simulink model "TosAeb"  (R2021a)
settings: Solver=FixedStepDiscrete  StartTime=0.0  StopTime=inf  FixedStep=0.01  RelTol=1e-3
undefined symbols (not defined in the file or in its folder): ACCModSts, AccSts1, AEB_ObjectMovingOrientation, AEB_ObjectMovingState, CN_BTCActive, CN_CurrentGearR, CN_CurrentGearR_DHT, CN_PTCActive, CN_VDCActive, CN_VSC_EspOff, CN_VSC_FCW_AEB_Error, CN_VSC_FCW_AEB_NoDispay, CN_VSC_FCW_AEB_PerfmDgdtn, CN_VSC_FCW_AEB_TempUnAvlbl, CN_VSC_VehStandstill, CNaccelAvoidMax, CNaccelAvoidMin, CNbrakePedalPress, CNcomfortStrLeftAccelMax, CNcomfortStrLeftAccelMin, CNcomfortStrRightAccelMax, CNcomfortStrRightAccelMin, CNDrvDoorOpen, CNDrvModeEco, CNDrvModeNorm, CNDrvModeOther, CNDrvModeSport, CNDrvSBRreminder, CNNull, CNobjInvalidID, CNPassDoorOpen, CNPassSBRreminder, CNPEBSAccelNegInf, CNPEBSAccelNull, CNPEBSTimeInf, CNPEBSTimeNull, CNSTOPMAX, CNSTOPNULL, CNsubjWidth, CNSwtOFF, CNSwtON, CNTTCMAX, CNTTCNULL, CNVehConfigAEBCycNone, CNVehConfigAEBNone, CNVehConfigAEBPedNone, CNVehConfigFCWNone, CNVehConfigJANone, CollisionSeverity, FALSE, FCWSnvty, JACycObjCalcInfoDefaultParams, JAPedObjCalcInfoDefaultParams, JAVehObjCalcInfoDefaultParams, latCycObjCalcInfoDefaultParams, latPedObjCalcInfoDefaultParams, longObjCalcInfoDefaultParams, ModSts, movingStatus, ObjectType ... (+1112)
libraries: simulink x3031, AEBSFunctionLib x50, Ta_Library x33, AEBSStateMachineLib x23, Mylibrary x12, simulink_extras x7, ObjSelect_lib x3, dspstat3 x2
(part 35 of 102)

== system TosAeb/FcwAebJa_Step_sys/ALG_AEBSystem/ALG_AEBController/AEB_VehicleStateCalcApp/VehSuppAborCondtionCalc/LatentWarningSuppAborConditonCalc/VehStateCalcLatentWarningSuppCalc ==
  AEB_VehResp : Inport
  FCW_AEB_VehFctSts : Inport  Port=2
  vehUnstableStateFlg : Inport  Port=3
  vehAssociatedSysStateSuppCond : Inport  Port=4
  "vehConfigFCW " : Inport  Port=5
  blockTimeWarning : Inport  Port=6
  Compare To Constant : simulink/Logic and Bit Operations/Compare To Constant  relop===  const=CNSwtOFF  OutDataTypeStr=boolean
  Compare To Constant1 : simulink/Logic and Bit Operations/Compare To Constant  relop===  const=CN_VSC_FCW_AEB_Error  OutDataTypeStr=boolean
  Compare To Constant2 : simulink/Logic and Bit Operations/Compare To Constant  relop===  const=CN_VSC_FCW_AEB_PerfmDgdtn  OutDataTypeStr=boolean
  Compare To Constant3 : simulink/Logic and Bit Operations/Compare To Constant  relop===  const=CNVehConfigFCWNone  OutDataTypeStr=boolean
  Compare To Constant4 : simulink/Logic and Bit Operations/Compare To Constant  relop=>  const=CNNull  OutDataTypeStr=boolean
  Compare To Constant5 : simulink/Logic and Bit Operations/Compare To Constant  relop===  const=TRUE  OutDataTypeStr=boolean
  Compare To Constant6 : simulink/Logic and Bit Operations/Compare To Constant  relop===  const=TRUE  OutDataTypeStr=boolean
  Compare To Constant7 : simulink/Logic and Bit Operations/Compare To Constant  relop===  const=CNSwtOFF  OutDataTypeStr=boolean
  Compare To Constant8 : simulink/Logic and Bit Operations/Compare To Constant  relop===  const=CN_VSC_FCW_AEB_TempUnAvlbl  OutDataTypeStr=boolean
  Constant : Constant  Value=P_AEB_InterSwt4LatentWarning
  Logical Operator : Logic  Operator=OR  Inputs=3  AllPortsSameDT=off  OutDataTypeStr=boolean
  Logical Operator1 : Logic  Operator=OR  Inputs=6  AllPortsSameDT=off  OutDataTypeStr=boolean
  Terminator : Terminator
  VSCvehLatentWarningSuppCond : Outport
  wires:
  Compare To Constant8/1 -> Logical Operator/3
  Compare To Constant1/1 -> Logical Operator/1
  Compare To Constant2/1 -> Logical Operator/2
  Compare To Constant7/1 -> Logical Operator1/6
  Constant/1 -> Compare To Constant7/1
  Compare To Constant6/1 -> Logical Operator1/3
  Compare To Constant5/1 -> Logical Operator1/4
  AEB_VehResp/1 -> Compare To Constant/1
  Compare To Constant/1 -> Logical Operator1/1
  Compare To Constant3/1 -> Logical Operator1/5
  vehAssociatedSysStateSuppCond/1 -> Compare To Constant5/1
  FCW_AEB_VehFctSts/1 -> Compare To Constant1/1, Compare To Constant8/1, Compare To Constant2/1
  blockTimeWarning/1 -> Compare To Constant4/1
  Logical Operator/1 -> Logical Operator1/2
  vehUnstableStateFlg/1 -> Compare To Constant6/1
  Logical Operator1/1 -> VSCvehLatentWarningSuppCond/1
  "vehConfigFCW "/1 -> Compare To Constant3/1
  Compare To Constant4/1 -> Terminator/1

== system TosAeb/FcwAebJa_Step_sys/ALG_AEBSystem/ALG_AEBController/AEB_VehicleStateCalcApp/VehSuppAborCondtionCalc/PreWarningSuppAborConditonCalc/VehStateCalcPreWarningAborCalc ==
  AEB_VehResp : Inport
  FCW_AEB_VehFctSts : Inport  Port=2
  vehAssociatedSysStateSuppCond : Inport  Port=3
  Compare To Constant : simulink/Logic and Bit Operations/Compare To Constant  relop===  const=CNSwtOFF  OutDataTypeStr=boolean
  Compare To Constant1 : simulink/Logic and Bit Operations/Compare To Constant  relop===  const=CN_VSC_FCW_AEB_Error  OutDataTypeStr=boolean
  Compare To Constant2 : simulink/Logic and Bit Operations/Compare To Constant  relop===  const=CN_VSC_FCW_AEB_PerfmDgdtn  OutDataTypeStr=boolean
  Compare To Constant3 : simulink/Logic and Bit Operations/Compare To Constant  relop===  const=TRUE  OutDataTypeStr=boolean
  Compare To Constant8 : simulink/Logic and Bit Operations/Compare To Constant  relop===  const=CN_VSC_FCW_AEB_TempUnAvlbl  OutDataTypeStr=boolean
  Logical Operator : Logic  Operator=OR  Inputs=3  AllPortsSameDT=off  OutDataTypeStr=boolean
  Logical Operator1 : Logic  Operator=OR  Inputs=3  AllPortsSameDT=off  OutDataTypeStr=boolean
  VSCvehPreWarningAborCond : Outport
  wires:
  Compare To Constant8/1 -> Logical Operator/3
  Compare To Constant1/1 -> Logical Operator/1
  Compare To Constant2/1 -> Logical Operator/2
  Compare To Constant3/1 -> Logical Operator1/3
  Logical Operator1/1 -> VSCvehPreWarningAborCond/1
  Logical Operator/1 -> Logical Operator1/2
  FCW_AEB_VehFctSts/1 -> Compare To Constant1/1, Compare To Constant2/1, Compare To Constant8/1
  vehAssociatedSysStateSuppCond/1 -> Compare To Constant3/1
  Compare To Constant/1 -> Logical Operator1/1
  AEB_VehResp/1 -> Compare To Constant/1

== system TosAeb/FcwAebJa_Step_sys/ALG_AEBSystem/ALG_AEBController/AEB_VehicleStateCalcApp/VehSuppAborCondtionCalc/PreWarningSuppAborConditonCalc/VehStateCalcPreWarningSuppCalc ==
  AEB_VehResp : Inport
  FCW_AEB_VehFctSts : Inport  Port=2
  vehUnstableStateFlg : Inport  Port=3
  vehAssociatedSysStateSuppCond : Inport  Port=4
  "vehConfigFCW " : Inport  Port=5
  blockTimeWarning : Inport  Port=6
  Compare To Constant : simulink/Logic and Bit Operations/Compare To Constant  relop===  const=CNSwtOFF  OutDataTypeStr=boolean
  Compare To Constant1 : simulink/Logic and Bit Operations/Compare To Constant  relop===  const=CN_VSC_FCW_AEB_Error  OutDataTypeStr=boolean
  Compare To Constant2 : simulink/Logic and Bit Operations/Compare To Constant  relop===  const=CN_VSC_FCW_AEB_PerfmDgdtn  OutDataTypeStr=boolean
  Compare To Constant3 : simulink/Logic and Bit Operations/Compare To Constant  relop===  const=CNVehConfigFCWNone  OutDataTypeStr=boolean
  Compare To Constant4 : simulink/Logic and Bit Operations/Compare To Constant  relop=>  const=CNNull  OutDataTypeStr=boolean
  Compare To Constant5 : simulink/Logic and Bit Operations/Compare To Constant  relop===  const=TRUE  OutDataTypeStr=boolean
  Compare To Constant6 : simulink/Logic and Bit Operations/Compare To Constant  relop===  const=TRUE  OutDataTypeStr=boolean
  Compare To Constant7 : simulink/Logic and Bit Operations/Compare To Constant  relop===  const=CNSwtOFF  OutDataTypeStr=boolean
  Compare To Constant8 : simulink/Logic and Bit Operations/Compare To Constant  relop===  const=CN_VSC_FCW_AEB_TempUnAvlbl  OutDataTypeStr=boolean
  Constant : Constant  Value=P_AEB_InterSwt4PreWarning
  Logical Operator : Logic  Operator=OR  Inputs=3  AllPortsSameDT=off  OutDataTypeStr=boolean
  Logical Operator1 : Logic  Operator=OR  Inputs=7  AllPortsSameDT=off  OutDataTypeStr=boolean
  VSCvehPreWarningSuppCond : Outport
  wires:
  Compare To Constant8/1 -> Logical Operator/3
  Compare To Constant7/1 -> Logical Operator1/7
  Constant/1 -> Compare To Constant7/1
  Compare To Constant6/1 -> Logical Operator1/3
  Compare To Constant5/1 -> Logical Operator1/4
  AEB_VehResp/1 -> Compare To Constant/1
  Compare To Constant/1 -> Logical Operator1/1
  Compare To Constant3/1 -> Logical Operator1/5
  vehAssociatedSysStateSuppCond/1 -> Compare To Constant5/1
  FCW_AEB_VehFctSts/1 -> Compare To Constant2/1, Compare To Constant8/1, Compare To Constant1/1
  blockTimeWarning/1 -> Compare To Constant4/1
  Logical Operator/1 -> Logical Operator1/2
  vehUnstableStateFlg/1 -> Compare To Constant6/1
  Logical Operator1/1 -> VSCvehPreWarningSuppCond/1
  "vehConfigFCW "/1 -> Compare To Constant3/1
  Compare To Constant2/1 -> Logical Operator/2
  Compare To Constant1/1 -> Logical Operator/1
  Compare To Constant4/1 -> Logical Operator1/6

== system TosAeb/FcwAebJa_Step_sys/ALG_AEBSystem/ALG_AEBController/AEB_VehicleStateCalcApp/VehSuppAborCondtionCalc/PrefillSuppAborConditonCalc/VehStateCalcPrefillAborCalc ==
  AEB_VehResp : Inport
  FCW_AEB_VehFctSts : Inport  Port=2
  vehUnstableStateFlg : Inport  Port=3
  vehAssociatedSysStateSuppCond : Inport  Port=4
  Compare To Constant : simulink/Logic and Bit Operations/Compare To Constant  relop===  const=CNSwtOFF  OutDataTypeStr=boolean
  Compare To Constant1 : simulink/Logic and Bit Operations/Compare To Constant  relop===  const=CN_VSC_FCW_AEB_Error  OutDataTypeStr=boolean
  Compare To Constant2 : simulink/Logic and Bit Operations/Compare To Constant  relop===  const=CN_VSC_FCW_AEB_PerfmDgdtn  OutDataTypeStr=boolean
  Compare To Constant3 : simulink/Logic and Bit Operations/Compare To Constant  relop===  const=TRUE  OutDataTypeStr=boolean
  Compare To Constant5 : simulink/Logic and Bit Operations/Compare To Constant  relop===  const=TRUE  OutDataTypeStr=boolean
  Compare To Constant8 : simulink/Logic and Bit Operations/Compare To Constant  relop===  const=CN_VSC_FCW_AEB_TempUnAvlbl  OutDataTypeStr=boolean
  Logical Operator : Logic  Operator=OR  Inputs=3  AllPortsSameDT=off  OutDataTypeStr=boolean
  Logical Operator1 : Logic  Operator=OR  Inputs=4  AllPortsSameDT=off  OutDataTypeStr=boolean
  VSCvehPrefillAborCond : Outport
  wires:
  Compare To Constant8/1 -> Logical Operator/3
  Compare To Constant1/1 -> Logical Operator/1
  Compare To Constant2/1 -> Logical Operator/2
  Compare To Constant3/1 -> Logical Operator1/4
  Compare To Constant5/1 -> Logical Operator1/3
  AEB_VehResp/1 -> Compare To Constant/1
  Compare To Constant/1 -> Logical Operator1/1
  vehAssociatedSysStateSuppCond/1 -> Compare To Constant3/1
  FCW_AEB_VehFctSts/1 -> Compare To Constant1/1, Compare To Constant2/1, Compare To Constant8/1
  Logical Operator/1 -> Logical Operator1/2
  vehUnstableStateFlg/1 -> Compare To Constant5/1
  Logical Operator1/1 -> VSCvehPrefillAborCond/1

== system TosAeb/FcwAebJa_Step_sys/ALG_AEBSystem/ALG_AEBController/AEB_VehicleStateCalcApp/VehSuppAborCondtionCalc/PrefillSuppAborConditonCalc/VehStateCalcPrefillSuppCalc ==
  AEB_VehResp : Inport
  FCW_AEB_VehFctSts : Inport  Port=2
  vehUnstableStateFlg : Inport  Port=3
  vehAssociatedSysStateSuppCond : Inport  Port=4
  "vehConfigAEB " : Inport  Port=5
  blockTimeBrakeReady : Inport  Port=6
  Compare To Constant : simulink/Logic and Bit Operations/Compare To Constant  relop===  const=CNSwtOFF  OutDataTypeStr=boolean
  Compare To Constant1 : simulink/Logic and Bit Operations/Compare To Constant  relop===  const=CN_VSC_FCW_AEB_Error  OutDataTypeStr=boolean
  Compare To Constant2 : simulink/Logic and Bit Operations/Compare To Constant  relop===  const=CN_VSC_FCW_AEB_PerfmDgdtn  OutDataTypeStr=boolean
  Compare To Constant3 : simulink/Logic and Bit Operations/Compare To Constant  relop===  const=CNVehConfigAEBNone  OutDataTypeStr=boolean
  Compare To Constant4 : simulink/Logic and Bit Operations/Compare To Constant  relop=>  const=CNNull  OutDataTypeStr=boolean
  Compare To Constant5 : simulink/Logic and Bit Operations/Compare To Constant  relop===  const=TRUE  OutDataTypeStr=boolean
  Compare To Constant6 : simulink/Logic and Bit Operations/Compare To Constant  relop===  const=TRUE  OutDataTypeStr=boolean
  Compare To Constant7 : simulink/Logic and Bit Operations/Compare To Constant  relop===  const=CNSwtOFF  OutDataTypeStr=boolean
  Compare To Constant8 : simulink/Logic and Bit Operations/Compare To Constant  relop===  const=CN_VSC_FCW_AEB_TempUnAvlbl  OutDataTypeStr=boolean
  Constant : Constant  Value=P_AEB_InterSwtSts4Prefill
  Logical Operator : Logic  Operator=OR  Inputs=3  AllPortsSameDT=off  OutDataTypeStr=boolean
  Logical Operator1 : Logic  Operator=OR  Inputs=6  AllPortsSameDT=off  OutDataTypeStr=boolean
  Terminator : Terminator
  VSCvehPrefillSuppCond : Outport
  wires:
  Compare To Constant8/1 -> Logical Operator/3
  Compare To Constant1/1 -> Logical Operator/1
  Compare To Constant2/1 -> Logical Operator/2
  Compare To Constant7/1 -> Logical Operator1/6
  Constant/1 -> Compare To Constant7/1
  Compare To Constant6/1 -> Logical Operator1/4
  Compare To Constant5/1 -> Logical Operator1/3
  Compare To Constant4/1 -> Terminator/1
  "vehConfigAEB "/1 -> Compare To Constant3/1
  Logical Operator1/1 -> VSCvehPrefillSuppCond/1
  vehUnstableStateFlg/1 -> Compare To Constant5/1
  Logical Operator/1 -> Logical Operator1/2
  blockTimeBrakeReady/1 -> Compare To Constant4/1
  FCW_AEB_VehFctSts/1 -> Compare To Constant1/1, Compare To Constant8/1, Compare To Constant2/1
  vehAssociatedSysStateSuppCond/1 -> Compare To Constant6/1
  Compare To Constant3/1 -> Logical Operator1/5
  Compare To Constant/1 -> Logical Operator1/1
  AEB_VehResp/1 -> Compare To Constant/1

== system TosAeb/FcwAebJa_Step_sys/ALG_AEBSystem/ALG_AEBInput/JaObjInfoMap/JaObjInfoMap/MovingStatus_Subsystem/InputMovingStatus ==
  note: 输出数值为3，类型为Moving
  note: 输出数值为2，类型为Stopped
  note: 输出数值为1，类型为Stationary
  note: 输出数值为0，类型为Unknown
  MovingStatus : Inport
  Compare To Constant : simulink/Logic and Bit Operations/Compare To Constant  relop===  const=0  OutDataTypeStr=boolean
  Compare To Constant1 : simulink/Logic and Bit Operations/Compare To Constant  relop===  const=1  OutDataTypeStr=boolean
  Compare To Constant2 : simulink/Logic and Bit Operations/Compare To Constant  relop===  const=3  OutDataTypeStr=boolean
  Compare To Constant8 : simulink/Logic and Bit Operations/Compare To Constant  relop===  const=2  OutDataTypeStr=boolean
  Input_Unknown : Outport
  Input_Stationary : Outport  Port=2
  Input_Stopped : Outport  Port=3
  Input_Moving : Outport  Port=4
  wires:
  Compare To Constant8/1 -> Input_Moving/1
  Compare To Constant1/1 -> Input_Stationary/1
  Compare To Constant2/1 -> Input_Stopped/1
  MovingStatus/1 -> Compare To Constant/1, Compare To Constant1/1, Compare To Constant2/1, Compare To Constant8/1
  Compare To Constant/1 -> Input_Unknown/1

== system TosAeb/FcwAebJa_Step_sys/ALG_AEBSystem/ALG_AEBInput/JaObjInfoMap/JaObjInfoMap/ObjType_Subsystem/InputObjectType ==
  note: 输出数值为2，类型为car
  note: 输出数值为3，类型为truck
  note: 输出数值为6，类型为pedestrian行人
  note: 输出数值为4，类型为motorbike摩托车
  note: 输出数值为5，类型为bicycle自行车
  note: 输出数值为7，类型为general_object
  note: 输出数值为1，类型为unknown
  u : Inport
  Compare To Constant : simulink/Logic and Bit Operations/Compare To Constant  relop===  const=0  OutDataTypeStr=boolean
  Compare To Constant1 : simulink/Logic and Bit Operations/Compare To Constant  relop===  const=1  OutDataTypeStr=boolean
  Compare To Constant10 : simulink/Logic and Bit Operations/Compare To Constant  relop===  const=7  OutDataTypeStr=boolean
  Compare To Constant11 : simulink/Logic and Bit Operations/Compare To Constant  relop===  const=9  OutDataTypeStr=boolean
  Compare To Constant12 : simulink/Logic and Bit Operations/Compare To Constant  relop===  const=8  OutDataTypeStr=boolean
  Compare To Constant13 : simulink/Logic and Bit Operations/Compare To Constant  relop===  const=15  OutDataTypeStr=boolean
  Compare To Constant14 : simulink/Logic and Bit Operations/Compare To Constant  relop===  const=10  OutDataTypeStr=boolean
  Compare To Constant15 : simulink/Logic and Bit Operations/Compare To Constant  relop===  const=11  OutDataTypeStr=boolean
  Compare To Constant16 : simulink/Logic and Bit Operations/Compare To Constant  relop===  const=12  OutDataTypeStr=boolean
  Compare To Constant17 : simulink/Logic and Bit Operations/Compare To Constant  relop===  const=14  OutDataTypeStr=boolean
  Compare To Constant18 : simulink/Logic and Bit Operations/Compare To Constant  relop===  const=12  OutDataTypeStr=boolean
  Compare To Constant2 : simulink/Logic and Bit Operations/Compare To Constant  relop===  const=16  OutDataTypeStr=boolean
  Compare To Constant3 : simulink/Logic and Bit Operations/Compare To Constant  relop===  const=2  OutDataTypeStr=boolean
  Compare To Constant4 : simulink/Logic and Bit Operations/Compare To Constant  relop===  const=3  OutDataTypeStr=boolean
  Compare To Constant5 : simulink/Logic and Bit Operations/Compare To Constant  relop===  const=4  OutDataTypeStr=boolean
  Compare To Constant6 : simulink/Logic and Bit Operations/Compare To Constant  relop===  const=5  OutDataTypeStr=boolean
  Compare To Constant7 : simulink/Logic and Bit Operations/Compare To Constant  relop===  const=18  OutDataTypeStr=boolean
  Compare To Constant8 : simulink/Logic and Bit Operations/Compare To Constant  relop===  const=17  OutDataTypeStr=boolean
  Compare To Constant9 : simulink/Logic and Bit Operations/Compare To Constant  relop===  const=6  OutDataTypeStr=boolean
  Logical Operator : Logic  Operator=OR  Inputs=4  AllPortsSameDT=off  OutDataTypeStr=boolean
  Logical Operator1 : Logic  Operator=OR  Inputs=5  AllPortsSameDT=off  OutDataTypeStr=boolean
  Logical Operator2 : Logic  Operator=OR  AllPortsSameDT=off  OutDataTypeStr=boolean
  Logical Operator3 : Logic  Operator=OR  AllPortsSameDT=off  OutDataTypeStr=boolean
  Logical Operator5 : Logic  Operator=OR  Inputs=4  AllPortsSameDT=off  OutDataTypeStr=boolean
  Input_ObjCar : Outport
  Input_ObjTruck : Outport  Port=2
  Input_ObjPed : Outport  Port=3
  Input_ObjMotor : Outport  Port=4
  Input_ObjBicycle : Outport  Port=5
  Input_ObjGeneral : Outport  Port=6
  Input_Unknown : Outport  Port=7
  wires:
  Compare To Constant12/1 -> Logical Operator3/1
  Compare To Constant6/1 -> Logical Operator1/4
  Logical Operator/1 -> Input_ObjCar/1
  Compare To Constant13/1 -> Logical Operator3/2
  u/1 -> Compare To Constant8/1, Compare To Constant18/1, Compare To Constant17/1, Compare To Constant16/1, Compare To Constant15/1, Compare To Constant14/1, Compare To Constant13/1, Compare To Constant12/1, Compare To Constant11/1, Compare To Constant10/1, Compare To Constant9/1, Compare To Constant7/1, Compare To Constant6/1, Compare To Constant5/1, Compare To Constant4/1, Compare To Constant3/1, Compare To Constant2/1, Compare To Constant1/1, Compare To Constant/1
  Compare To Constant2/1 -> Logical Operator/3
  Compare To Constant1/1 -> Logical Operator/2
  Compare To Constant16/1 -> Logical Operator5/3
  Compare To Constant18/1 -> Input_Unknown/1
  Compare To Constant15/1 -> Logical Operator5/2
  Compare To Constant10/1 -> Logical Operator2/1
  Logical Operator5/1 -> Input_ObjGeneral/1
  Compare To Constant5/1 -> Logical Operator1/3
  Logical Operator3/1 -> Input_ObjBicycle/1
  Logical Operator1/1 -> Input_ObjTruck/1
  Compare To Constant14/1 -> Logical Operator5/1
  Compare To Constant3/1 -> Logical Operator1/1
  Logical Operator2/1 -> Input_ObjMotor/1
  Compare To Constant7/1 -> Logical Operator1/5
  Compare To Constant4/1 -> Logical Operator1/2
  Compare To Constant11/1 -> Logical Operator2/2
  Compare To Constant8/1 -> Logical Operator/4
  Compare To Constant/1 -> Logical Operator/1
  Compare To Constant17/1 -> Logical Operator5/4
  Compare To Constant9/1 -> Input_ObjPed/1

== system TosAeb/FcwAebJa_Step_sys/ALG_AEBSystem/ALG_AEBInput/JaObjInfoMap/JaObjInfoMap/Subsystem/MovingStatus_Subsystem ==
  Alg_MovingStatus : Inport
  InputMovingStatus : Subsystem  TreatAsAtomicUnit=on
  MovingStatusSwitch : Stateflow chart  TreatAsAtomicUnit=on
  MovingStatusOut : Outport
  wires:
  Alg_MovingStatus/1 -> InputMovingStatus/1(MovingStatus)
  MovingStatusSwitch/1(MovingStatus) -> MovingStatusOut/1
  InputMovingStatus/1(Input_Unknown) -> MovingStatusSwitch/1(Input_Unknown)
  InputMovingStatus/2(Input_Stationary) -> MovingStatusSwitch/2(Input_Stationary)
  InputMovingStatus/3(Input_Stopped) -> MovingStatusSwitch/3(Input_Stopped)
  InputMovingStatus/4(Input_Moving) -> MovingStatusSwitch/4(Input_Moving)

== system TosAeb/FcwAebJa_Step_sys/ALG_AEBSystem/ALG_AEBInput/JaObjInfoMap/JaObjInfoMap/Subsystem/ObjType_Subsystem1 ==
  Alg_ObjType : Inport
  InputObjectType : Subsystem  TreatAsAtomicUnit=on
  ObjectType : Stateflow chart  TreatAsAtomicUnit=on
  ObjType : Outport
  wires:
  InputObjectType/4(Input_ObjMotor) -> ObjectType/4(ObjMotor)
  InputObjectType/6(Input_ObjGeneral) -> ObjectType/6(ObjGeneral)
  InputObjectType/3(Input_ObjPed) -> ObjectType/3(ObjPed)
  InputObjectType/7(Input_Unknown) -> ObjectType/7(Unknown)
  InputObjectType/5(Input_ObjBicycle) -> ObjectType/5(ObjBicycle)
  InputObjectType/2(Input_ObjTruck) -> ObjectType/2(ObjTruck)
  InputObjectType/1(Input_ObjCar) -> ObjectType/1(ObjCar)
  ObjectType/1(ObjType) -> ObjType/1
  Alg_ObjType/1 -> InputObjectType/1(InputObjType)

== system TosAeb/FcwAebJa_Step_sys/ALG_AEBSystem/ALG_AEBInput/JaObjInfoMap/JaObjInfoMap/Subsystem1/MovingStatus_Subsystem ==
  Alg_MovingStatus : Inport
  InputMovingStatus : Subsystem  TreatAsAtomicUnit=on
  MovingStatusSwitch : Stateflow chart  TreatAsAtomicUnit=on
  MovingStatusOut : Outport
  wires:
  Alg_MovingStatus/1 -> InputMovingStatus/1(MovingStatus)
  MovingStatusSwitch/1(MovingStatus) -> MovingStatusOut/1
  InputMovingStatus/1(Input_Unknown) -> MovingStatusSwitch/1(Input_Unknown)
  InputMovingStatus/2(Input_Stationary) -> MovingStatusSwitch/2(Input_Stationary)
  InputMovingStatus/3(Input_Stopped) -> MovingStatusSwitch/3(Input_Stopped)
  InputMovingStatus/4(Input_Moving) -> MovingStatusSwitch/4(Input_Moving)

== system TosAeb/FcwAebJa_Step_sys/ALG_AEBSystem/ALG_AEBInput/JaObjInfoMap/JaObjInfoMap/Subsystem1/ObjType_Subsystem1 ==
  Alg_ObjType : Inport
  InputObjectType : Subsystem  TreatAsAtomicUnit=on
  ObjectType : Stateflow chart  TreatAsAtomicUnit=on
  ObjType : Outport
  wires:
  InputObjectType/4(Input_ObjMotor) -> ObjectType/4(ObjMotor)
  InputObjectType/6(Input_ObjGeneral) -> ObjectType/6(ObjGeneral)
  InputObjectType/3(Input_ObjPed) -> ObjectType/3(ObjPed)
  InputObjectType/7(Input_Unknown) -> ObjectType/7(Unknown)
  InputObjectType/5(Input_ObjBicycle) -> ObjectType/5(ObjBicycle)
  InputObjectType/2(Input_ObjTruck) -> ObjectType/2(ObjTruck)
  InputObjectType/1(Input_ObjCar) -> ObjectType/1(ObjCar)
  ObjectType/1(ObjType) -> ObjType/1
  Alg_ObjType/1 -> InputObjectType/1(InputObjType)

== system TosAeb/FcwAebJa_Step_sys/ALG_AEBSystem/ALG_AEBInput/LatObjInfoMap/latObjInfoMap/MovingStatus_Subsystem/InputMovingStatus ==
  note: 输出数值为3，类型为Moving
  note: 输出数值为2，类型为Stopped
  note: 输出数值为1，类型为Stationary
  note: 输出数值为0，类型为Unknown
  MovingStatus : Inport
  Compare To Constant : simulink/Logic and Bit Operations/Compare To Constant  relop===  const=0  OutDataTypeStr=boolean
  Compare To Constant1 : simulink/Logic and Bit Operations/Compare To Constant  relop===  const=1  OutDataTypeStr=boolean
  Compare To Constant2 : simulink/Logic and Bit Operations/Compare To Constant  relop===  const=3  OutDataTypeStr=boolean
  Compare To Constant8 : simulink/Logic and Bit Operations/Compare To Constant  relop===  const=2  OutDataTypeStr=boolean
  Input_Unknown : Outport
  Input_Stationary : Outport  Port=2
  Input_Stopped : Outport  Port=3
  Input_Moving : Outport  Port=4
  wires:
  Compare To Constant8/1 -> Input_Moving/1
  Compare To Constant1/1 -> Input_Stationary/1
  Compare To Constant2/1 -> Input_Stopped/1
  MovingStatus/1 -> Compare To Constant/1, Compare To Constant1/1, Compare To Constant2/1, Compare To Constant8/1
  Compare To Constant/1 -> Input_Unknown/1

== system TosAeb/FcwAebJa_Step_sys/ALG_AEBSystem/ALG_AEBInput/LatObjInfoMap/latObjInfoMap/ObjType_Subsystem/InputObjectType ==
  note: 输出数值为2，类型为car
  note: 输出数值为3，类型为truck
  note: 输出数值为6，类型为pedestrian行人
  note: 输出数值为4，类型为motorbike摩托车
  note: 输出数值为5，类型为bicycle自行车
  note: 输出数值为7，类型为general_object
  note: 输出数值为1，类型为unknown
  u : Inport
  Compare To Constant : simulink/Logic and Bit Operations/Compare To Constant  relop===  const=0  OutDataTypeStr=boolean
  Compare To Constant1 : simulink/Logic and Bit Operations/Compare To Constant  relop===  const=1  OutDataTypeStr=boolean
  Compare To Constant10 : simulink/Logic and Bit Operations/Compare To Constant  relop===  const=7  OutDataTypeStr=boolean
  Compare To Constant11 : simulink/Logic and Bit Operations/Compare To Constant  relop===  const=9  OutDataTypeStr=boolean
  Compare To Constant12 : simulink/Logic and Bit Operations/Compare To Constant  relop===  const=8  OutDataTypeStr=boolean
  Compare To Constant13 : simulink/Logic and Bit Operations/Compare To Constant  relop===  const=15  OutDataTypeStr=boolean
  Compare To Constant14 : simulink/Logic and Bit Operations/Compare To Constant  relop===  const=10  OutDataTypeStr=boolean
  Compare To Constant15 : simulink/Logic and Bit Operations/Compare To Constant  relop===  const=11  OutDataTypeStr=boolean
  Compare To Constant16 : simulink/Logic and Bit Operations/Compare To Constant  relop===  const=12  OutDataTypeStr=boolean
  Compare To Constant17 : simulink/Logic and Bit Operations/Compare To Constant  relop===  const=14  OutDataTypeStr=boolean
  Compare To Constant18 : simulink/Logic and Bit Operations/Compare To Constant  relop===  const=12  OutDataTypeStr=boolean
  Compare To Constant2 : simulink/Logic and Bit Operations/Compare To Constant  relop===  const=16  OutDataTypeStr=boolean
  Compare To Constant3 : simulink/Logic and Bit Operations/Compare To Constant  relop===  const=2  OutDataTypeStr=boolean
  Compare To Constant4 : simulink/Logic and Bit Operations/Compare To Constant  relop===  const=3  OutDataTypeStr=boolean
  Compare To Constant5 : simulink/Logic and Bit Operations/Compare To Constant  relop===  const=4  OutDataTypeStr=boolean
  Compare To Constant6 : simulink/Logic and Bit Operations/Compare To Constant  relop===  const=5  OutDataTypeStr=boolean
  Compare To Constant7 : simulink/Logic and Bit Operations/Compare To Constant  relop===  const=18  OutDataTypeStr=boolean
  Compare To Constant8 : simulink/Logic and Bit Operations/Compare To Constant  relop===  const=17  OutDataTypeStr=boolean
  Compare To Constant9 : simulink/Logic and Bit Operations/Compare To Constant  relop===  const=6  OutDataTypeStr=boolean
  Logical Operator : Logic  Operator=OR  Inputs=4  AllPortsSameDT=off  OutDataTypeStr=boolean
  Logical Operator1 : Logic  Operator=OR  Inputs=5  AllPortsSameDT=off  OutDataTypeStr=boolean
  Logical Operator2 : Logic  Operator=OR  AllPortsSameDT=off  OutDataTypeStr=boolean
  Logical Operator3 : Logic  Operator=OR  AllPortsSameDT=off  OutDataTypeStr=boolean
  Logical Operator5 : Logic  Operator=OR  Inputs=4  AllPortsSameDT=off  OutDataTypeStr=boolean
  Input_ObjCar : Outport
  Input_ObjTruck : Outport  Port=2
  Input_ObjPed : Outport  Port=3
  Input_ObjMotor : Outport  Port=4
  Input_ObjBicycle : Outport  Port=5
  Input_ObjGeneral : Outport  Port=6
  Input_Unknown : Outport  Port=7
  wires:
  Compare To Constant12/1 -> Logical Operator3/1
  Compare To Constant6/1 -> Logical Operator1/4
  Logical Operator/1 -> Input_ObjCar/1
  Compare To Constant13/1 -> Logical Operator3/2
  u/1 -> Compare To Constant8/1, Compare To Constant18/1, Compare To Constant17/1, Compare To Constant16/1, Compare To Constant15/1, Compare To Constant14/1, Compare To Constant13/1, Compare To Constant12/1, Compare To Constant11/1, Compare To Constant10/1, Compare To Constant9/1, Compare To Constant7/1, Compare To Constant6/1, Compare To Constant5/1, Compare To Constant4/1, Compare To Constant3/1, Compare To Constant2/1, Compare To Constant1/1, Compare To Constant/1
  Compare To Constant2/1 -> Logical Operator/3
  Compare To Constant1/1 -> Logical Operator/2
  Compare To Constant16/1 -> Logical Operator5/3
  Compare To Constant18/1 -> Input_Unknown/1
  Compare To Constant15/1 -> Logical Operator5/2
  Compare To Constant10/1 -> Logical Operator2/1
  Logical Operator5/1 -> Input_ObjGeneral/1
  Compare To Constant5/1 -> Logical Operator1/3
  Logical Operator3/1 -> Input_ObjBicycle/1
  Logical Operator1/1 -> Input_ObjTruck/1
  Compare To Constant14/1 -> Logical Operator5/1
  Compare To Constant3/1 -> Logical Operator1/1
  Logical Operator2/1 -> Input_ObjMotor/1
  Compare To Constant7/1 -> Logical Operator1/5
  Compare To Constant4/1 -> Logical Operator1/2
  Compare To Constant11/1 -> Logical Operator2/2
  Compare To Constant8/1 -> Logical Operator/4
  Compare To Constant/1 -> Logical Operator/1
  Compare To Constant17/1 -> Logical Operator5/4
  Compare To Constant9/1 -> Input_ObjPed/1

== system TosAeb/FcwAebJa_Step_sys/ALG_AEBSystem/ALG_AEBInput/LatObjInfoMap/latObjInfoMap/Subsystem/MovingStatus_Subsystem ==
  Alg_MovingStatus : Inport
  InputMovingStatus : Subsystem  TreatAsAtomicUnit=on
  MovingStatusSwitch : Stateflow chart  TreatAsAtomicUnit=on
  MovingStatusOut : Outport
  wires:
  Alg_MovingStatus/1 -> InputMovingStatus/1(MovingStatus)
  MovingStatusSwitch/1(MovingStatus) -> MovingStatusOut/1
  InputMovingStatus/1(Input_Unknown) -> MovingStatusSwitch/1(Input_Unknown)
  InputMovingStatus/2(Input_Stationary) -> MovingStatusSwitch/2(Input_Stationary)
  InputMovingStatus/3(Input_Stopped) -> MovingStatusSwitch/3(Input_Stopped)
  InputMovingStatus/4(Input_Moving) -> MovingStatusSwitch/4(Input_Moving)

== system TosAeb/FcwAebJa_Step_sys/ALG_AEBSystem/ALG_AEBInput/LatObjInfoMap/latObjInfoMap/Subsystem/ObjType_Subsystem1 ==
  Alg_ObjType : Inport
  InputObjectType : Subsystem  TreatAsAtomicUnit=on
  ObjectType : Stateflow chart  TreatAsAtomicUnit=on
  ObjType : Outport
  wires:
  InputObjectType/4(Input_ObjMotor) -> ObjectType/4(ObjMotor)
  InputObjectType/6(Input_ObjGeneral) -> ObjectType/6(ObjGeneral)
  InputObjectType/3(Input_ObjPed) -> ObjectType/3(ObjPed)
  InputObjectType/7(Input_Unknown) -> ObjectType/7(Unknown)
  InputObjectType/5(Input_ObjBicycle) -> ObjectType/5(ObjBicycle)
  InputObjectType/2(Input_ObjTruck) -> ObjectType/2(ObjTruck)
  InputObjectType/1(Input_ObjCar) -> ObjectType/1(ObjCar)
  ObjectType/1(ObjType) -> ObjType/1
  Alg_ObjType/1 -> InputObjectType/1(InputObjType)

== system TosAeb/FcwAebJa_Step_sys/ALG_AEBSystem/ALG_AEBInput/LatObjInfoMap/latObjInfoMap/Subsystem1/MovingStatus_Subsystem ==
  Alg_MovingStatus : Inport
  InputMovingStatus : Subsystem  TreatAsAtomicUnit=on
  MovingStatusSwitch : Stateflow chart  TreatAsAtomicUnit=on
  MovingStatusOut : Outport
  wires:
  Alg_MovingStatus/1 -> InputMovingStatus/1(MovingStatus)
  MovingStatusSwitch/1(MovingStatus) -> MovingStatusOut/1
  InputMovingStatus/1(Input_Unknown) -> MovingStatusSwitch/1(Input_Unknown)
  InputMovingStatus/2(Input_Stationary) -> MovingStatusSwitch/2(Input_Stationary)
  InputMovingStatus/3(Input_Stopped) -> MovingStatusSwitch/3(Input_Stopped)
  InputMovingStatus/4(Input_Moving) -> MovingStatusSwitch/4(Input_Moving)

== system TosAeb/FcwAebJa_Step_sys/ALG_AEBSystem/ALG_AEBInput/LatObjInfoMap/latObjInfoMap/Subsystem1/ObjType_Subsystem1 ==
  Alg_ObjType : Inport
  InputObjectType : Subsystem  TreatAsAtomicUnit=on
  ObjectType : Stateflow chart  TreatAsAtomicUnit=on
  ObjType : Outport
  wires:
  InputObjectType/4(Input_ObjMotor) -> ObjectType/4(ObjMotor)
  InputObjectType/6(Input_ObjGeneral) -> ObjectType/6(ObjGeneral)
  InputObjectType/3(Input_ObjPed) -> ObjectType/3(ObjPed)
  InputObjectType/7(Input_Unknown) -> ObjectType/7(Unknown)
  InputObjectType/5(Input_ObjBicycle) -> ObjectType/5(ObjBicycle)
  InputObjectType/2(Input_ObjTruck) -> ObjectType/2(ObjTruck)
  InputObjectType/1(Input_ObjCar) -> ObjectType/1(ObjCar)
  ObjectType/1(ObjType) -> ObjType/1
  Alg_ObjType/1 -> InputObjectType/1(InputObjType)

== system TosAeb/FcwAebJa_Step_sys/ALG_AEBSystem/ALG_AEBInput/LongObjInfoMap/Subsystem/MovingStatus_Subsystem/InputMovingStatus ==
  note: 输出数值为3，类型为Moving
  note: 输出数值为2，类型为Stopped
  note: 输出数值为1，类型为Stationary
  note: 输出数值为0，类型为Unknown
  MovingStatus : Inport
  Compare To Constant : simulink/Logic and Bit Operations/Compare To Constant  relop===  const=0  OutDataTypeStr=boolean
  Compare To Constant1 : simulink/Logic and Bit Operations/Compare To Constant  relop===  const=1  OutDataTypeStr=boolean
  Compare To Constant2 : simulink/Logic and Bit Operations/Compare To Constant  relop===  const=3  OutDataTypeStr=boolean
  Compare To Constant8 : simulink/Logic and Bit Operations/Compare To Constant  relop===  const=2  OutDataTypeStr=boolean
  Input_Unknown : Outport
  Input_Stationary : Outport  Port=2
  Input_Stopped : Outport  Port=3
  Input_Moving : Outport  Port=4
  wires:
  Compare To Constant8/1 -> Input_Moving/1
  Compare To Constant1/1 -> Input_Stationary/1
  Compare To Constant2/1 -> Input_Stopped/1
  MovingStatus/1 -> Compare To Constant/1, Compare To Constant1/1, Compare To Constant2/1, Compare To Constant8/1
  Compare To Constant/1 -> Input_Unknown/1

== system TosAeb/FcwAebJa_Step_sys/ALG_AEBSystem/ALG_AEBInput/LongObjInfoMap/Subsystem/ObjType_Subsystem/InputObjectType ==
  note: 输出数值为2，类型为car
  note: 输出数值为3，类型为truck
  note: 输出数值为6，类型为pedestrian行人
  note: 输出数值为4，类型为motorbike摩托车
  note: 输出数值为5，类型为bicycle自行车
  note: 输出数值为7，类型为general_object
  note: 输出数值为1，类型为unknown
  InputObjType : Inport
  Compare To Constant : simulink/Logic and Bit Operations/Compare To Constant  relop===  const=0  OutDataTypeStr=boolean
  Compare To Constant1 : simulink/Logic and Bit Operations/Compare To Constant  relop===  const=1  OutDataTypeStr=boolean
  Compare To Constant10 : simulink/Logic and Bit Operations/Compare To Constant  relop===  const=7  OutDataTypeStr=boolean
  Compare To Constant11 : simulink/Logic and Bit Operations/Compare To Constant  relop===  const=9  OutDataTypeStr=boolean
  Compare To Constant12 : simulink/Logic and Bit Operations/Compare To Constant  relop===  const=8  OutDataTypeStr=boolean
  Compare To Constant13 : simulink/Logic and Bit Operations/Compare To Constant  relop===  const=15  OutDataTypeStr=boolean
  Compare To Constant14 : simulink/Logic and Bit Operations/Compare To Constant  relop===  const=10  OutDataTypeStr=boolean
  Compare To Constant15 : simulink/Logic and Bit Operations/Compare To Constant  relop===  const=11  OutDataTypeStr=boolean
  Compare To Constant16 : simulink/Logic and Bit Operations/Compare To Constant  relop===  const=12  OutDataTypeStr=boolean
  Compare To Constant17 : simulink/Logic and Bit Operations/Compare To Constant  relop===  const=14  OutDataTypeStr=boolean
  Compare To Constant18 : simulink/Logic and Bit Operations/Compare To Constant  relop===  const=12  OutDataTypeStr=boolean
  Compare To Constant2 : simulink/Logic and Bit Operations/Compare To Constant  relop===  const=16  OutDataTypeStr=boolean
  Compare To Constant3 : simulink/Logic and Bit Operations/Compare To Constant  relop===  const=2  OutDataTypeStr=boolean
  Compare To Constant4 : simulink/Logic and Bit Operations/Compare To Constant  relop===  const=3  OutDataTypeStr=boolean
  Compare To Constant5 : simulink/Logic and Bit Operations/Compare To Constant  relop===  const=4  OutDataTypeStr=boolean
  Compare To Constant6 : simulink/Logic and Bit Operations/Compare To Constant  relop===  const=5  OutDataTypeStr=boolean
  Compare To Constant7 : simulink/Logic and Bit Operations/Compare To Constant  relop===  const=18  OutDataTypeStr=boolean
  Compare To Constant8 : simulink/Logic and Bit Operations/Compare To Constant  relop===  const=17  OutDataTypeStr=boolean
  Compare To Constant9 : simulink/Logic and Bit Operations/Compare To Constant  relop===  const=6  OutDataTypeStr=boolean
  Logical Operator : Logic  Operator=OR  Inputs=4  AllPortsSameDT=off  OutDataTypeStr=boolean
  Logical Operator1 : Logic  Operator=OR  Inputs=5  AllPortsSameDT=off  OutDataTypeStr=boolean
  Logical Operator2 : Logic  Operator=OR  AllPortsSameDT=off  OutDataTypeStr=boolean
  Logical Operator3 : Logic  Operator=OR  AllPortsSameDT=off  OutDataTypeStr=boolean
  Logical Operator5 : Logic  Operator=OR  Inputs=4  AllPortsSameDT=off  OutDataTypeStr=boolean
  Input_ObjCar : Outport
  Input_ObjTruck : Outport  Port=2
  Input_ObjPed : Outport  Port=3
  Input_ObjMotor : Outport  Port=4
  Input_ObjBicycle : Outport  Port=5
  Input_ObjGeneral : Outport  Port=6
  Input_Unknown : Outport  Port=7
  wires:
  Compare To Constant12/1 -> Logical Operator3/1
  Compare To Constant6/1 -> Logical Operator1/4
  Logical Operator/1 -> Input_ObjCar/1
  Compare To Constant13/1 -> Logical Operator3/2
  InputObjType/1 -> Compare To Constant8/1, Compare To Constant18/1, Compare To Constant17/1, Compare To Constant16/1, Compare To Constant15/1, Compare To Constant14/1, Compare To Constant13/1, Compare To Constant12/1, Compare To Constant11/1, Compare To Constant10/1, Compare To Constant9/1, Compare To Constant7/1, Compare To Constant6/1, Compare To Constant5/1, Compare To Constant4/1, Compare To Constant3/1, Compare To Constant2/1, Compare To Constant1/1, Compare To Constant/1
  Compare To Constant2/1 -> Logical Operator/3
  Compare To Constant1/1 -> Logical Operator/2
  Compare To Constant16/1 -> Logical Operator5/3
  Compare To Constant18/1 -> Input_Unknown/1
  Compare To Constant15/1 -> Logical Operator5/2
  Compare To Constant10/1 -> Logical Operator2/1
  Logical Operator5/1 -> Input_ObjGeneral/1
  Compare To Constant5/1 -> Logical Operator1/3
  Logical Operator3/1 -> Input_ObjBicycle/1
  Logical Operator1/1 -> Input_ObjTruck/1
  Compare To Constant14/1 -> Logical Operator5/1
  Compare To Constant3/1 -> Logical Operator1/1
  Logical Operator2/1 -> Input_ObjMotor/1
  Compare To Constant7/1 -> Logical Operator1/5
  Compare To Constant4/1 -> Logical Operator1/2
  Compare To Constant11/1 -> Logical Operator2/2
  Compare To Constant8/1 -> Logical Operator/4
  Compare To Constant/1 -> Logical Operator/1
  Compare To Constant17/1 -> Logical Operator5/4
  Compare To Constant9/1 -> Input_ObjPed/1

== system TosAeb/FcwAebJa_Step_sys/ALG_AEBSystem/ALG_AEBInput/LongObjInfoMap/Subsystem1/MovingStatus_Subsystem/InputMovingStatus ==
  note: 输出数值为3，类型为Moving
  note: 输出数值为2，类型为Stopped
  note: 输出数值为1，类型为Stationary
  note: 输出数值为0，类型为Unknown
  MovingStatus : Inport
  Compare To Constant : simulink/Logic and Bit Operations/Compare To Constant  relop===  const=0  OutDataTypeStr=boolean
  Compare To Constant1 : simulink/Logic and Bit Operations/Compare To Constant  relop===  const=1  OutDataTypeStr=boolean
  Compare To Constant2 : simulink/Logic and Bit Operations/Compare To Constant  relop===  const=3  OutDataTypeStr=boolean
  Compare To Constant8 : simulink/Logic and Bit Operations/Compare To Constant  relop===  const=2  OutDataTypeStr=boolean
  Input_Unknown : Outport
  Input_Stationary : Outport  Port=2
  Input_Stopped : Outport  Port=3
  Input_Moving : Outport  Port=4
  wires:
  Compare To Constant8/1 -> Input_Moving/1
  Compare To Constant1/1 -> Input_Stationary/1
  Compare To Constant2/1 -> Input_Stopped/1
  MovingStatus/1 -> Compare To Constant/1, Compare To Constant1/1, Compare To Constant2/1, Compare To Constant8/1
  Compare To Constant/1 -> Input_Unknown/1

== system TosAeb/FcwAebJa_Step_sys/ALG_AEBSystem/ALG_AEBInput/LongObjInfoMap/Subsystem1/ObjType_Subsystem1/InputObjectType ==
  note: 输出数值为2，类型为car
  note: 输出数值为3，类型为truck
  note: 输出数值为6，类型为pedestrian行人
  note: 输出数值为4，类型为motorbike摩托车
  note: 输出数值为5，类型为bicycle自行车
  note: 输出数值为7，类型为general_object
  note: 输出数值为1，类型为unknown
  InputObjType : Inport
  Compare To Constant : simulink/Logic and Bit Operations/Compare To Constant  relop===  const=0  OutDataTypeStr=boolean
  Compare To Constant1 : simulink/Logic and Bit Operations/Compare To Constant  relop===  const=1  OutDataTypeStr=boolean
  Compare To Constant10 : simulink/Logic and Bit Operations/Compare To Constant  relop===  const=7  OutDataTypeStr=boolean
  Compare To Constant11 : simulink/Logic and Bit Operations/Compare To Constant  relop===  const=9  OutDataTypeStr=boolean
  Compare To Constant12 : simulink/Logic and Bit Operations/Compare To Constant  relop===  const=8  OutDataTypeStr=boolean
  Compare To Constant13 : simulink/Logic and Bit Operations/Compare To Constant  relop===  const=15  OutDataTypeStr=boolean
  Compare To Constant14 : simulink/Logic and Bit Operations/Compare To Constant  relop===  const=10  OutDataTypeStr=boolean
  Compare To Constant15 : simulink/Logic and Bit Operations/Compare To Constant  relop===  const=11  OutDataTypeStr=boolean
  Compare To Constant16 : simulink/Logic and Bit Operations/Compare To Constant  relop===  const=12  OutDataTypeStr=boolean
  Compare To Constant17 : simulink/Logic and Bit Operations/Compare To Constant  relop===  const=14  OutDataTypeStr=boolean
  Compare To Constant18 : simulink/Logic and Bit Operations/Compare To Constant  relop===  const=12  OutDataTypeStr=boolean
  Compare To Constant2 : simulink/Logic and Bit Operations/Compare To Constant  relop===  const=16  OutDataTypeStr=boolean
  Compare To Constant3 : simulink/Logic and Bit Operations/Compare To Constant  relop===  const=2  OutDataTypeStr=boolean
  Compare To Constant4 : simulink/Logic and Bit Operations/Compare To Constant  relop===  const=3  OutDataTypeStr=boolean
  Compare To Constant5 : simulink/Logic and Bit Operations/Compare To Constant  relop===  const=4  OutDataTypeStr=boolean
  Compare To Constant6 : simulink/Logic and Bit Operations/Compare To Constant  relop===  const=5  OutDataTypeStr=boolean
  Compare To Constant7 : simulink/Logic and Bit Operations/Compare To Constant  relop===  const=18  OutDataTypeStr=boolean
  Compare To Constant8 : simulink/Logic and Bit Operations/Compare To Constant  relop===  const=17  OutDataTypeStr=boolean
  Compare To Constant9 : simulink/Logic and Bit Operations/Compare To Constant  relop===  const=6  OutDataTypeStr=boolean
  Logical Operator : Logic  Operator=OR  Inputs=4  AllPortsSameDT=off  OutDataTypeStr=boolean
  Logical Operator1 : Logic  Operator=OR  Inputs=5  AllPortsSameDT=off  OutDataTypeStr=boolean
  Logical Operator2 : Logic  Operator=OR  AllPortsSameDT=off  OutDataTypeStr=boolean
  Logical Operator3 : Logic  Operator=OR  AllPortsSameDT=off  OutDataTypeStr=boolean
  Logical Operator5 : Logic  Operator=OR  Inputs=4  AllPortsSameDT=off  OutDataTypeStr=boolean
  Input_ObjCar : Outport
  Input_ObjTruck : Outport  Port=2
  Input_ObjPed : Outport  Port=3
  Input_ObjMotor : Outport  Port=4
  Input_ObjBicycle : Outport  Port=5
  Input_ObjGeneral : Outport  Port=6
  Input_Unknown : Outport  Port=7
  wires:
  Compare To Constant12/1 -> Logical Operator3/1
  Compare To Constant6/1 -> Logical Operator1/4
  Logical Operator/1 -> Input_ObjCar/1
  Compare To Constant13/1 -> Logical Operator3/2
  InputObjType/1 -> Compare To Constant8/1, Compare To Constant18/1, Compare To Constant17/1, Compare To Constant16/1, Compare To Constant15/1, Compare To Constant14/1, Compare To Constant13/1, Compare To Constant12/1, Compare To Constant11/1, Compare To Constant10/1, Compare To Constant9/1, Compare To Constant7/1, Compare To Constant6/1, Compare To Constant5/1, Compare To Constant4/1, Compare To Constant3/1, Compare To Constant2/1, Compare To Constant1/1, Compare To Constant/1
  Compare To Constant2/1 -> Logical Operator/3
  Compare To Constant1/1 -> Logical Operator/2
  Compare To Constant16/1 -> Logical Operator5/3
  Compare To Constant18/1 -> Input_Unknown/1
  Compare To Constant15/1 -> Logical Operator5/2
  Compare To Constant10/1 -> Logical Operator2/1
  Logical Operator5/1 -> Input_ObjGeneral/1
  Compare To Constant5/1 -> Logical Operator1/3
  Logical Operator3/1 -> Input_ObjBicycle/1
  Logical Operator1/1 -> Input_ObjTruck/1
  Compare To Constant14/1 -> Logical Operator5/1
  Compare To Constant3/1 -> Logical Operator1/1
  Logical Operator2/1 -> Input_ObjMotor/1
  Compare To Constant7/1 -> Logical Operator1/5
  Compare To Constant4/1 -> Logical Operator1/2
  Compare To Constant11/1 -> Logical Operator2/2
  Compare To Constant8/1 -> Logical Operator/4
  Compare To Constant/1 -> Logical Operator/1
  Compare To Constant17/1 -> Logical Operator5/4
  Compare To Constant9/1 -> Input_ObjPed/1

== system TosAeb/FcwAebJa_Step_sys/ALG_AEBSystem/ALG_AEBOutput/AEBS_DVRInfoCalc/AEB_JAPED_DVR_INFO/Subsystem/AEBObjStateMap4 ==
  ObjectMovingState : Inport
  ObjectMovingOrientation : Inport  Port=2
  AEBObjStateFlow : Stateflow chart  TreatAsAtomicUnit=on
  AEBObjState : Outport
  wires:
  ObjectMovingOrientation/1 -> AEBObjStateFlow/2(ObjectMovingOrientation)
  ObjectMovingState/1 -> AEBObjStateFlow/1(ObjectMovingState)
  AEBObjStateFlow/1(AEBObjState) -> AEBObjState/1

== system TosAeb/FcwAebJa_Step_sys/ALG_AEBSystem/ALG_AEBOutput/AEBS_DVRInfoCalc/AEB_JAVEH_DVR_INFO/Subsystem/AEBObjStateMap ==
  ObjectMovingState : Inport
  ObjectMovingOrientation : Inport  Port=2
  AEBObjStateFlow : Stateflow chart  TreatAsAtomicUnit=on
  AEBObjState : Outport
  wires:
  AEBObjStateFlow/1(AEBObjState) -> AEBObjState/1
  ObjectMovingState/1 -> AEBObjStateFlow/1(ObjectMovingState)
  ObjectMovingOrientation/1 -> AEBObjStateFlow/2(ObjectMovingOrientation)

== system TosAeb/FcwAebJa_Step_sys/ALG_AEBSystem/ALG_AEBOutput/AEBS_DVRInfoCalc/AEB_LATCYC_DVR_INFO/Subsystem/AEBObjStateMap ==
  ObjectMovingState : Inport
  ObjectMovingOrientation : Inport  Port=2
  AEBObjStateFlow : Stateflow chart  TreatAsAtomicUnit=on
  AEBObjState : Outport
  wires:
  ObjectMovingOrientation/1 -> AEBObjStateFlow/2(ObjectMovingOrientation)
  ObjectMovingState/1 -> AEBObjStateFlow/1(ObjectMovingState)
  AEBObjStateFlow/1(AEBObjState) -> AEBObjState/1

== system TosAeb/FcwAebJa_Step_sys/ALG_AEBSystem/ALG_AEBOutput/AEBS_DVRInfoCalc/AEB_LATPED_DVR_INFO/Subsystem/AEBObjStateMap ==
  ObjectMovingState : Inport
  ObjectMovingOrientation : Inport  Port=2
  AEBObjStateFlow : Stateflow chart  TreatAsAtomicUnit=on
  AEBObjState : Outport
  wires:
  AEBObjStateFlow/1(AEBObjState) -> AEBObjState/1
  ObjectMovingState/1 -> AEBObjStateFlow/1(ObjectMovingState)
  ObjectMovingOrientation/1 -> AEBObjStateFlow/2(ObjectMovingOrientation)

== system TosAeb/FcwAebJa_Step_sys/ALG_AEBSystem/ALG_AEBOutput/AEBS_DVRInfoCalc/AEB_LON_DVR_INFO/If Action Subsystem/AEBObjStateMap ==
  ObjectMovingState : Inport
  ObjectMovingOrientation : Inport  Port=2
  AEBObjStateFlow : Stateflow chart  TreatAsAtomicUnit=on
  AEBObjState : Outport
  wires:
  ObjectMovingOrientation/1 -> AEBObjStateFlow/2(ObjectMovingOrientation)
  ObjectMovingState/1 -> AEBObjStateFlow/1(ObjectMovingState)
  AEBObjStateFlow/1(AEBObjState) -> AEBObjState/1

== system TosAeb/AEB_Objsel/TA_InfoRebuild/TAObjInfoCalc/For Each Subsystem/Info_rebuild/Road_relative/LineInfo_Process/LineInfo_Process_Left ==
  Leftline_Data : Inport
  Bus Creator : BusCreator  Inputs=7
  Bus Selector : BusSelector  OutputSignals=lineSegNum,lineSegs
  Bus Selector1 : BusSelector  OutputSignals=lineSegValid,lineSegStart,lineSegEnd,lineC0,lineC1,lineC2,lineC3
  Bus Selector2 : BusSelector  OutputSignals=lineSegValid,lineSegStart,lineSegEnd,lineC0,lineC1,lineC2,lineC3
  Bus Selector3 : BusSelector  OutputSignals=lineSegValid,lineSegStart,lineSegEnd,lineC0,lineC1,lineC2,lineC3
  Data Type Conversion : DataTypeConversion  OutDataTypeStr=boolean
  Data Type Conversion1 : DataTypeConversion  OutDataTypeStr=boolean
  Data Type Conversion2 : DataTypeConversion  OutDataTypeStr=boolean
  Line_Info : MATLAB Function  TreatAsAtomicUnit=on
  Selector18 : Selector  IndexMode=Zero-based  InputPortWidth=3  IndexOptions=Index vector (dialog)  Indices=[0]  OutputSizes=1
  Selector2 : Selector  IndexMode=Zero-based  InputPortWidth=3  IndexOptions=Index vector (dialog)  Indices=[1]  OutputSizes=1
  Selector3 : Selector  IndexMode=Zero-based  InputPortWidth=3  IndexOptions=Index vector (dialog)  Indices=[2]  OutputSizes=1
  LeftLine_Info : Outport
  wires:
  Bus Selector/1 -> Line_Info/1(seg_num)  "<lineSegNum>"
  Bus Selector3/7 -> Line_Info/22(seg3_C3)  "<lineC3>"
  Bus Selector3/6 -> Line_Info/21(seg3_C2)  "<lineC2>"
  Bus Selector3/5 -> Line_Info/20(seg3_C1)  "<lineC1>"
  Bus Selector3/4 -> Line_Info/19(seg3_C0)  "<lineC0>"
  Bus Selector3/3 -> Line_Info/18(seg3_end)  "<lineSegEnd>"
  Bus Selector3/2 -> Line_Info/17(seg3_Start)  "<lineSegStart>"
  Bus Selector3/1 -> Data Type Conversion2/1  "<lineSegValid>"
  Bus Selector2/7 -> Line_Info/15(seg2_C3)  "<lineC3>"
  Bus Selector2/6 -> Line_Info/14(seg2_C2)  "<lineC2>"
  Bus Selector2/5 -> Line_Info/13(seg2_C1)  "<lineC1>"
  Bus Selector2/4 -> Line_Info/12(seg2_C0)  "<lineC0>"
  Bus Selector2/3 -> Line_Info/11(seg2_end)  "<lineSegEnd>"
  Bus Selector2/2 -> Line_Info/10(seg2_Start)  "<lineSegStart>"
  Bus Selector2/1 -> Data Type Conversion1/1  "<lineSegValid>"
  Bus Selector1/7 -> Line_Info/8(seg1_C3)  "<lineC3>"
  Bus Selector1/6 -> Line_Info/7(seg1_C2)  "<lineC2>"
  Bus Selector1/5 -> Line_Info/6(seg1_C1)  "<lineC1>"
  Bus Selector1/4 -> Line_Info/5(seg1_C0)  "<lineC0>"
  Bus Selector1/3 -> Line_Info/4(seg1_end)  "<lineSegEnd>"
  Bus Selector1/2 -> Line_Info/3(seg1_Start)  "<lineSegStart>"
  Bus Selector1/1 -> Data Type Conversion/1  "<lineSegValid>"
  Bus Selector/2 -> Selector18/1, Selector2/1, Selector3/1  "<lineSegs>"
  Selector2/1 -> Bus Selector2/1
  Selector18/1 -> Bus Selector1/1
  Selector3/1 -> Bus Selector3/1
  Leftline_Data/1 -> Bus Selector/1
  Line_Info/1(Valid) -> Bus Creator/1
  Line_Info/2(Line_start) -> Bus Creator/2
  Line_Info/3(Line_end) -> Bus Creator/3
  Line_Info/4(C0) -> Bus Creator/4
  Line_Info/5(C1) -> Bus Creator/5
  Line_Info/6(C2) -> Bus Creator/6
  Line_Info/7(C3) -> Bus Creator/7
  Bus Creator/1 -> LeftLine_Info/1
  Data Type Conversion/1 -> Line_Info/2(seg1_valid)
  Data Type Conversion1/1 -> Line_Info/9(seg2_valid)
  Data Type Conversion2/1 -> Line_Info/16(seg3_valid)
